simulink model "VirtualADAPT"  (Simulink 8.9)
settings: Solver=ode45  StartTime=0.0  StopTime=inf  RelTol=1e-3  EnableMultiTasking=on
PostLoadFcn:
  | FaultInjectionGUIHandle = FaultInjectionGUI;
InitFcn:
  | VirtualADAPTDataStructure
undefined symbols (not defined in the file or in its folder): ln
libraries: simulink x363
(part 20 of 26)

== system VirtualADAPT/VirtualADAPTv1/PowerStorage/Battery1Subsystem/SensorE142/R/Computing R ==
  In1 : Inport
  RNominal : Inport  Port=2
  Fault Profile : Inport  Port=3
  Step : Inport  Port=4
  Ramp : Inport  Port=5
  JuncDB : Inport  Port=6
  Bond : Inport  Port=7
  Junction Type : Inport  Port=8
  CalcCausality : Fcn  Expr=(u(1) == u(2) && u(3) ==1) || (u(1) !=u(2) && u(3) == 0)
  CalcOutput : Fcn  Expr=(u(1)*u(2)^u(3))
  MinusOne : Constant  Value=-1
  Mux1 : Mux  Inputs=3
  Mux2 : Mux  Inputs=3
  One : Constant
  Switch1 : MultiPortSwitch  SaturateOnIntegerOverflow=on  InputSameDT=on
  Switch2 : MultiPortSwitch  DataPortOrder=Zero-based contiguous  Inputs=2  zeroidx=on  SaturateOnIntegerOverflow=on  InputSameDT=on
  Out1 : Outport
  Out2 : Outport  Port=2
  wires:
  In1/1 -> Mux2/1
  Fault Profile/1 -> Switch1/1
  RNominal/1 -> Switch1/2
  Step/1 -> Switch1/3
  Ramp/1 -> Switch1/4
  Switch1/1 -> Out2/1, Mux2/2
  JuncDB/1 -> Mux1/1
  Bond/1 -> Mux1/2
  Junction Type/1 -> Mux1/3
  Mux1/1 -> CalcCausality/1
  CalcCausality/1 -> Switch2/1
  One/1 -> Switch2/2
  MinusOne/1 -> Switch2/3
  Switch2/1 -> Mux2/3
  Mux2/1 -> CalcOutput/1
  CalcOutput/1 -> Out1/1

== system VirtualADAPT/VirtualADAPTv1/PowerStorage/Battery1Subsystem/SensorISH136/MSe/Fault Inject ==
  Nominal : Inport
  Fault Profile : Inport  Port=2
  Step : Inport  Port=3
  Ramp : Inport  Port=4
  Bias : Inport  Port=5
  stuckAt : Inport  Port=6
  Switch1 : MultiPortSwitch  Inputs=5  SaturateOnIntegerOverflow=on  InputSameDT=on
  Out1 : Outport
  wires:
  Nominal/1 -> Switch1/2
  Fault Profile/1 -> Switch1/1
  Step/1 -> Switch1/3
  Ramp/1 -> Switch1/4
  Bias/1 -> Switch1/5
  stuckAt/1 -> Switch1/6
  Switch1/1 -> Out1/1

== system VirtualADAPT/VirtualADAPTv1/PowerStorage/Battery1Subsystem/SensorISH136/R/Computing R ==
  In1 : Inport
  RNominal : Inport  Port=2
  Fault Profile : Inport  Port=3
  Step : Inport  Port=4
  Ramp : Inport  Port=5
  JuncDB : Inport  Port=6
  Bond : Inport  Port=7
  Junction Type : Inport  Port=8
  CalcCausality : Fcn  Expr=(u(1) == u(2) && u(3) ==1) || (u(1) !=u(2) && u(3) == 0)
  CalcOutput : Fcn  Expr=(u(1)*u(2)^u(3))
  MinusOne : Constant  Value=-1
  Mux1 : Mux  Inputs=3
  Mux2 : Mux  Inputs=3
  One : Constant
  Switch1 : MultiPortSwitch  SaturateOnIntegerOverflow=on  InputSameDT=on
  Switch2 : MultiPortSwitch  DataPortOrder=Zero-based contiguous  Inputs=2  zeroidx=on  SaturateOnIntegerOverflow=on  InputSameDT=on
  Out1 : Outport
  Out2 : Outport  Port=2
  wires:
  In1/1 -> Mux2/1
  Fault Profile/1 -> Switch1/1
  RNominal/1 -> Switch1/2
  Step/1 -> Switch1/3
  Ramp/1 -> Switch1/4
  Switch1/1 -> Out2/1, Mux2/2
  JuncDB/1 -> Mux1/1
  Bond/1 -> Mux1/2
  Junction Type/1 -> Mux1/3
  Mux1/1 -> CalcCausality/1
  CalcCausality/1 -> Switch2/1
  One/1 -> Switch2/2
  MinusOne/1 -> Switch2/3
  Switch2/1 -> Mux2/3
  Mux2/1 -> CalcOutput/1
  CalcOutput/1 -> Out1/1

== system VirtualADAPT/VirtualADAPTv1/PowerStorage/Battery1Subsystem/SensorIT140/MSe/Fault Inject ==
  Nominal : Inport
  Fault Profile : Inport  Port=2
  Step : Inport  Port=3
  Ramp : Inport  Port=4
  Bias : Inport  Port=5
  stuckAt : Inport  Port=6
  Switch1 : MultiPortSwitch  Inputs=5  SaturateOnIntegerOverflow=on  InputSameDT=on
  Out1 : Outport
  wires:
  Nominal/1 -> Switch1/2
  Fault Profile/1 -> Switch1/1
  Step/1 -> Switch1/3
  Ramp/1 -> Switch1/4
  Bias/1 -> Switch1/5
  stuckAt/1 -> Switch1/6
  Switch1/1 -> Out1/1

== system VirtualADAPT/VirtualADAPTv1/PowerStorage/Battery1Subsystem/SensorIT140/R/Computing R ==
  In1 : Inport
  RNominal : Inport  Port=2
  Fault Profile : Inport  Port=3
  Step : Inport  Port=4
  Ramp : Inport  Port=5
  JuncDB : Inport  Port=6
  Bond : Inport  Port=7
  Junction Type : Inport  Port=8
  CalcCausality : Fcn  Expr=(u(1) == u(2) && u(3) ==1) || (u(1) !=u(2) && u(3) == 0)
  CalcOutput : Fcn  Expr=(u(1)*u(2)^u(3))
  MinusOne : Constant  Value=-1
  Mux1 : Mux  Inputs=3
  Mux2 : Mux  Inputs=3
  One : Constant
  Switch1 : MultiPortSwitch  SaturateOnIntegerOverflow=on  InputSameDT=on
  Switch2 : MultiPortSwitch  DataPortOrder=Zero-based contiguous  Inputs=2  zeroidx=on  SaturateOnIntegerOverflow=on  InputSameDT=on
  Out1 : Outport
  Out2 : Outport  Port=2
  wires:
  In1/1 -> Mux2/1
  Fault Profile/1 -> Switch1/1
  RNominal/1 -> Switch1/2
  Step/1 -> Switch1/3
  Ramp/1 -> Switch1/4
  Switch1/1 -> Out2/1, Mux2/2
  JuncDB/1 -> Mux1/1
  Bond/1 -> Mux1/2
  Junction Type/1 -> Mux1/3
  Mux1/1 -> CalcCausality/1
  CalcCausality/1 -> Switch2/1
  One/1 -> Switch2/2
  MinusOne/1 -> Switch2/3
  Switch2/1 -> Mux2/3
  Mux2/1 -> CalcOutput/1
  CalcOutput/1 -> Out1/1

== system VirtualADAPT/VirtualADAPTv1/PowerStorage/Battery1Subsystem/SensorTE128/MSe/Fault Inject ==
  Nominal : Inport
  Fault Profile : Inport  Port=2
  Step : Inport  Port=3
  Ramp : Inport  Port=4
  Bias : Inport  Port=5
  stuckAt : Inport  Port=6
  Switch1 : MultiPortSwitch  Inputs=5  SaturateOnIntegerOverflow=on  InputSameDT=on
  Out1 : Outport
  wires:
  Nominal/1 -> Switch1/2
  Fault Profile/1 -> Switch1/1
  Step/1 -> Switch1/3
  Ramp/1 -> Switch1/4
  Bias/1 -> Switch1/5
  stuckAt/1 -> Switch1/6
  Switch1/1 -> Out1/1

== system VirtualADAPT/VirtualADAPTv1/PowerStorage/Battery1Subsystem/SensorTE128/R/Computing R ==
  In1 : Inport
  RNominal : Inport  Port=2
  Fault Profile : Inport  Port=3
  Step : Inport  Port=4
  Ramp : Inport  Port=5
  JuncDB : Inport  Port=6
  Bond : Inport  Port=7
  Junction Type : Inport  Port=8
  CalcCausality : Fcn  Expr=(u(1) == u(2) && u(3) ==1) || (u(1) !=u(2) && u(3) == 0)
  CalcOutput : Fcn  Expr=(u(1)*u(2)^u(3))
  MinusOne : Constant  Value=-1
  Mux1 : Mux  Inputs=3
  Mux2 : Mux  Inputs=3
  One : Constant
  Switch1 : MultiPortSwitch  SaturateOnIntegerOverflow=on  InputSameDT=on
  Switch2 : MultiPortSwitch  DataPortOrder=Zero-based contiguous  Inputs=2  zeroidx=on  SaturateOnIntegerOverflow=on  InputSameDT=on
  Out1 : Outport
  Out2 : Outport  Port=2
  wires:
  In1/1 -> Mux2/1
  Fault Profile/1 -> Switch1/1
  RNominal/1 -> Switch1/2
  Step/1 -> Switch1/3
  Ramp/1 -> Switch1/4
  Switch1/1 -> Out2/1, Mux2/2
  JuncDB/1 -> Mux1/1
  Bond/1 -> Mux1/2
  Junction Type/1 -> Mux1/3
  Mux1/1 -> CalcCausality/1
  CalcCausality/1 -> Switch2/1
  One/1 -> Switch2/2
  MinusOne/1 -> Switch2/3
  Switch2/1 -> Mux2/3
  Mux2/1 -> CalcOutput/1
  CalcOutput/1 -> Out1/1

== system VirtualADAPT/VirtualADAPTv1/PowerStorage/Battery1Subsystem/SensorTE128/TempBias/Fault Inject ==
  Nominal : Inport
  Fault Profile : Inport  Port=2
  Step : Inport  Port=3
  Ramp : Inport  Port=4
  Bias : Inport  Port=5
  stuckAt : Inport  Port=6
  Switch1 : MultiPortSwitch  Inputs=5  SaturateOnIntegerOverflow=on  InputSameDT=on
  Out1 : Outport
  wires:
  Nominal/1 -> Switch1/2
  Fault Profile/1 -> Switch1/1
  Step/1 -> Switch1/3
  Ramp/1 -> Switch1/4
  Bias/1 -> Switch1/5
  stuckAt/1 -> Switch1/6
  Switch1/1 -> Out1/1

== system VirtualADAPT/VirtualADAPTv1/PowerStorage/Battery1Subsystem/SensorTE129/MSe/Fault Inject ==
  Nominal : Inport
  Fault Profile : Inport  Port=2
  Step : Inport  Port=3
  Ramp : Inport  Port=4
  Bias : Inport  Port=5
  stuckAt : Inport  Port=6
  Switch1 : MultiPortSwitch  Inputs=5  SaturateOnIntegerOverflow=on  InputSameDT=on
  Out1 : Outport
  wires:
  Nominal/1 -> Switch1/2
  Fault Profile/1 -> Switch1/1
  Step/1 -> Switch1/3
  Ramp/1 -> Switch1/4
  Bias/1 -> Switch1/5
  stuckAt/1 -> Switch1/6
  Switch1/1 -> Out1/1

== system VirtualADAPT/VirtualADAPTv1/PowerStorage/Battery1Subsystem/SensorTE129/R/Computing R ==
  In1 : Inport
  RNominal : Inport  Port=2
  Fault Profile : Inport  Port=3
  Step : Inport  Port=4
  Ramp : Inport  Port=5
  JuncDB : Inport  Port=6
  Bond : Inport  Port=7
  Junction Type : Inport  Port=8
  CalcCausality : Fcn  Expr=(u(1) == u(2) && u(3) ==1) || (u(1) !=u(2) && u(3) == 0)
  CalcOutput : Fcn  Expr=(u(1)*u(2)^u(3))
  MinusOne : Constant  Value=-1
  Mux1 : Mux  Inputs=3
  Mux2 : Mux  Inputs=3
  One : Constant
  Switch1 : MultiPortSwitch  SaturateOnIntegerOverflow=on  InputSameDT=on
  Switch2 : MultiPortSwitch  DataPortOrder=Zero-based contiguous  Inputs=2  zeroidx=on  SaturateOnIntegerOverflow=on  InputSameDT=on
  Out1 : Outport
  Out2 : Outport  Port=2
  wires:
  In1/1 -> Mux2/1
  Fault Profile/1 -> Switch1/1
  RNominal/1 -> Switch1/2
  Step/1 -> Switch1/3
  Ramp/1 -> Switch1/4
  Switch1/1 -> Out2/1, Mux2/2
  JuncDB/1 -> Mux1/1
  Bond/1 -> Mux1/2
  Junction Type/1 -> Mux1/3
  Mux1/1 -> CalcCausality/1
  CalcCausality/1 -> Switch2/1
  One/1 -> Switch2/2
  MinusOne/1 -> Switch2/3
  Switch2/1 -> Mux2/3
  Mux2/1 -> CalcOutput/1
  CalcOutput/1 -> Out1/1

== system VirtualADAPT/VirtualADAPTv1/PowerStorage/Battery1Subsystem/SensorTE129/TempBias/Fault Inject ==
  Nominal : Inport
  Fault Profile : Inport  Port=2
  Step : Inport  Port=3
  Ramp : Inport  Port=4
  Bias : Inport  Port=5
  stuckAt : Inport  Port=6
  Switch1 : MultiPortSwitch  Inputs=5  SaturateOnIntegerOverflow=on  InputSameDT=on
  Out1 : Outport
  wires:
  Nominal/1 -> Switch1/2
  Fault Profile/1 -> Switch1/1
  Step/1 -> Switch1/3
  Ramp/1 -> Switch1/4
  Bias/1 -> Switch1/5
  stuckAt/1 -> Switch1/6
  Switch1/1 -> Out1/1

== system VirtualADAPT/VirtualADAPTv1/PowerStorage/Battery2Subsystem/Battery2/Charge/C ==
  F : Inport
  Capacitance : Constant  Value=C
  Fault Inject : Subsystem
  Fault Profile : Constant  Value=FP
  InitialCondition : Constant  Value=IC
  Integrator : Integrator  InitialConditionSource=external
  Product1 : Product  SaturateOnIntegerOverflow=on  RndMeth=Zero  InputSameDT=on  OutDataTypeStr=Inherit: Same as first input
  Product2 : Product  SaturateOnIntegerOverflow=on  RndMeth=Zero  InputSameDT=on  OutDataTypeStr=Inherit: Same as first input
  Ramp : simulink/Sources/Ramp  slope=FM  start=FT  X0=C
  Reciprocal : Math  Operator=reciprocal  SaturateOnIntegerOverflow=on
  Step : Step  Time=FT  Before=C  After=(1 + FM)*C  SampleTime=0
  E : Outport
  C : Outport  Port=2
  q : Outport  Port=3
  wires:
  F/1 -> Integrator/1
  InitialCondition/1 -> Product1/1
  Capacitance/1 -> Fault Inject/1(Nominal), Product1/2
  Product1/1 -> Integrator/2
  Integrator/1 -> Product2/1, q/1
  Fault Inject/1(Out1) -> Reciprocal/1, C/1
  Reciprocal/1 -> Product2/2
  Fault Profile/1 -> Fault Inject/2(Fault Profile)
  Step/1 -> Fault Inject/3(Step)
  Ramp/1 -> Fault Inject/4(Ramp)
  Product2/1 -> E/1

== system VirtualADAPT/VirtualADAPTv1/PowerStorage/Battery2Subsystem/Battery2/Charge/ComputeDOC ==
  u(1) : Inport
  u(2) : Inport  Port=2
  u(3) : Inport  Port=3
  Function : Fcn  Expr=1-(2765360-u(1))/(1.33*270720*(1-u(3)/(-35))^0.642/(1+(1.33-1)*(u(2)/5)^0.61))
  Mux : Mux  Inputs=3
  y(1) : Outport
  wires:
  u(1)/1 -> Mux/1
  u(2)/1 -> Mux/2
  u(3)/1 -> Mux/3
  Mux/1 -> Function/1
  Function/1 -> y(1)/1

== system VirtualADAPT/VirtualADAPTv1/PowerStorage/Battery2Subsystem/Battery2/Charge/ComputeSOC ==
  u(1) : Inport
  u(2) : Inport  Port=2
  Function : Fcn  Expr=1-(2765360-u(2))/(1.33*270720*(1-u(1)/(-35))^0.642)
  Mux : Mux  Inputs=2
  y(1) : Outport
  wires:
  u(1)/1 -> Mux/1
  u(2)/1 -> Mux/2
  Mux/1 -> Function/1
  Function/1 -> y(1)/1

== system VirtualADAPT/VirtualADAPTv1/PowerStorage/Battery2Subsystem/Battery2/Charge/Function ==
  u(1) : Inport
  Function : Fcn  Expr=u(1)
  Mux : Mux  Inputs=1
  y(1) : Outport
  wires:
  u(1)/1 -> Mux/1
  Mux/1 -> Function/1
  Function/1 -> y(1)/1

== system VirtualADAPT/VirtualADAPTv1/PowerStorage/Battery2Subsystem/Battery2/Charge/IbattNegative ==
  u(1) : Inport
  Function : Fcn  Expr=u(1)<0
  Mux : Mux  Inputs=1
  One : Constant
  Switch : Switch  Criteria=u2 ~= 0  SaturateOnIntegerOverflow=on  InputSameDT=on
  Zero : Constant  Value=0
  y(1) : Outport
  wires:
  u(1)/1 -> Mux/1
  Mux/1 -> Function/1
  Function/1 -> Switch/2
  One/1 -> Switch/1
  Zero/1 -> Switch/3
  Switch/1 -> y(1)/1

== system VirtualADAPT/VirtualADAPTv1/PowerStorage/Battery2Subsystem/Battery2/Charge/Imod ==
  u(1) : Inport
  u(2) : Inport  Port=2
  u(3) : Inport  Port=3
  Adder : Sum  Inputs=-  SaturateOnIntegerOverflow=on  InputSameDT=on  OutDataTypeStr=Inherit: Same as first input
  Gain : Gain  Gain=-1  SaturateOnIntegerOverflow=on  OutDataTypeStr=Inherit: Same as input  ParamDataTypeStr=Inherit: Same as input
  Mux : Mux  Inputs=3
  OffGuard : Fcn  Expr=(u(3))
  OnGuard : Fcn  Expr=(!u(3))
  Product1 : Product  SaturateOnIntegerOverflow=on  RndMeth=Zero  InputSameDT=on  OutDataTypeStr=Inherit: Same as first input
  Product2 : Product  SaturateOnIntegerOverflow=on  RndMeth=Zero  InputSameDT=on  OutDataTypeStr=Inherit: Same as first input
  determineState : Subsystem
  y(1) : Outport
  y(2) : Outport  Port=2
  O : Outport  Port=3
  State : Outport  Port=4
  wires:
  Adder/1 -> Gain/1
  u(3)/1 -> Mux/3
  Mux/1 -> OnGuard/1, OffGuard/1
  OnGuard/1 -> determineState/1(In1)
  OffGuard/1 -> determineState/2(In2)
  u(2)/1 -> Product1/1
  determineState/1(Out1) -> Product1/2, Product2/2, State/1
  Product1/1 -> O/1, y(1)/1
  Gain/1 -> Product2/1
  u(1)/1 -> Adder/1
  Product2/1 -> y(2)/1

== system VirtualADAPT/VirtualADAPTv1/PowerStorage/Battery2Subsystem/Battery2/Charge/Sf ==
  E : Inport
  Sf : Inport  Port=2
  Bias : Step  Time=FT  After=FM  SampleTime=0
  Fault Inject : Subsystem
  Fault Profile : Constant  Value=FP
  Mult2 : Product  SaturateOnIntegerOverflow=on  RndMeth=Zero  InputSameDT=on  OutDataTypeStr=Inherit: Same as first input
  Multiply : Product  SaturateOnIntegerOverflow=on  RndMeth=Zero  InputSameDT=on  OutDataTypeStr=Inherit: Same as first input
  Ramp : simulink/Sources/Ramp  slope=FM  start=FT  X0=0
  Step : Step  Time=FT  Before=1  After=(1 + FM)  SampleTime=0
  StuckAt : Step  Time=FT  Before=1  After=0  SampleTime=0
  StuckAt2 : Step  Time=FT  After=FM  SampleTime=0
  Sum : Sum  SaturateOnIntegerOverflow=on  InputSameDT=on  OutDataTypeStr=Inherit: Same as first input
  Sum2 : Sum  SaturateOnIntegerOverflow=on  InputSameDT=on  OutDataTypeStr=Inherit: Same as first input
  Sum3 : Sum  SaturateOnIntegerOverflow=on  InputSameDT=on  OutDataTypeStr=Inherit: Same as first input
  F : Outport
  wires:
  Fault Inject/1(Out1) -> F/1
  Sf/1 -> Fault Inject/1(Nominal), Multiply/1, Sum/1, Sum2/1, Mult2/1
  Fault Profile/1 -> Fault Inject/2(Fault Profile)
  Step/1 -> Multiply/2
  Ramp/1 -> Sum/2
  Multiply/1 -> Fault Inject/3(Step)
  Sum/1 -> Fault Inject/4(Ramp)
  Bias/1 -> Sum2/2
  Sum2/1 -> Fault Inject/5(Bias)
  StuckAt/1 -> Mult2/2
  Mult2/1 -> Sum3/1
  StuckAt2/1 -> Sum3/2
  Sum3/1 -> Fault Inject/6(stuckAt)

== system VirtualADAPT/VirtualADAPTv1/PowerStorage/Battery2Subsystem/Battery2/Charge/ZeroJunction ==
  u(1) : Inport
  u(2) : Inport  Port=2
  Adder : Sum  Inputs=+  SaturateOnIntegerOverflow=on  InputSameDT=on  OutDataTypeStr=Inherit: Same as first input
  Gain : Gain  SaturateOnIntegerOverflow=on  OutDataTypeStr=Inherit: Same as input  ParamDataTypeStr=Inherit: Same as input
  y(1) : Outport
  y(2) : Outport  Port=2
  O : Outport  Port=3
  wires:
  Adder/1 -> Gain/1
  u(2)/1 -> y(1)/1, O/1
  u(1)/1 -> Adder/1
  Gain/1 -> y(2)/1

== system VirtualADAPT/VirtualADAPTv1/PowerStorage/Battery2Subsystem/Battery2/CircuitEquivalent/BatteryConnected ==
  Constant : Constant  Value=Constant
  Fault Inject : Subsystem
  Fault Profile : Constant  Value=FP
  Ramp : simulink/Sources/Ramp  slope=FM  start=FT  X0=Constant
  Step : Step  Time=FT  Before=Constant  After=(1 + FM)*Constant  SampleTime=0
  C : Outport
  wires:
  Fault Inject/1(Out1) -> C/1
  Constant/1 -> Fault Inject/1(Nominal)
  Fault Profile/1 -> Fault Inject/2(Fault Profile)
  Step/1 -> Fault Inject/3(Step)
  Ramp/1 -> Fault Inject/4(Ramp)

== system VirtualADAPT/VirtualADAPTv1/PowerStorage/Battery2Subsystem/Battery2/CircuitEquivalent/Bug ==
  u(1) : Inport
  u(2) : Inport  Port=2
  Adder : Sum  Inputs=+  SaturateOnIntegerOverflow=on  InputSameDT=on  OutDataTypeStr=Inherit: Same as first input
  Gain : Gain  SaturateOnIntegerOverflow=on  OutDataTypeStr=Inherit: Same as input  ParamDataTypeStr=Inherit: Same as input
  y(1) : Outport
  y(2) : Outport  Port=2
  O : Outport  Port=3
  wires:
  Adder/1 -> Gain/1
  Gain/1 -> y(1)/1
  u(1)/1 -> y(2)/1, O/1
  u(2)/1 -> Adder/1

== system VirtualADAPT/VirtualADAPTv1/PowerStorage/Battery2Subsystem/Battery2/CircuitEquivalent/C0 ==
  F : Inport
  Capacitance : Constant  Value=C
  Data Type Conversion : DataTypeConversion  OutDataTypeStr=boolean
  Detect Change : simulink/Logic and Bit Operations/Detect Change  vinit=0  InputProcessing=Inherited  OutDataTypeStr=uint8
  Fault Inject : Subsystem
  Fault Profile : Constant  Value=FP
  InitialCondition : Constant  Value=IC
  Integrator : Integrator  ExternalReset=either  InitialConditionSource=external
  Product1 : Product  SaturateOnIntegerOverflow=on  RndMeth=Zero  InputSameDT=on  OutDataTypeStr=Inherit: Same as first input
  Product2 : Product  SaturateOnIntegerOverflow=on  RndMeth=Zero  InputSameDT=on  OutDataTypeStr=Inherit: Same as first input
  Ramp : simulink/Sources/Ramp  slope=FM  start=FT  X0=C
  Reciprocal : Math  Operator=reciprocal  SaturateOnIntegerOverflow=on
  Step : Step  Time=FT  Before=C  After=(1 + FM)*C  SampleTime=0
  E : Outport
  C : Outport  Port=2
  q : Outport  Port=3
  wires:
  F/1 -> Integrator/1
  InitialCondition/1 -> Product1/1, Detect Change/1
  Capacitance/1 -> Fault Inject/1(Nominal), Product1/2
  Product1/1 -> Integrator/3
  Integrator/1 -> Product2/1, q/1
  Fault Inject/1(Out1) -> Reciprocal/1, C/1
  Reciprocal/1 -> Product2/2
  Fault Profile/1 -> Fault Inject/2(Fault Profile)
  Step/1 -> Fault Inject/3(Step)
  Ramp/1 -> Fault Inject/4(Ramp)
  Product2/1 -> E/1
  Detect Change/1 -> Data Type Conversion/1
  Data Type Conversion/1 -> Integrator/2

== system VirtualADAPT/VirtualADAPTv1/PowerStorage/Battery2Subsystem/Battery2/CircuitEquivalent/C1 ==
  F : Inport
  Capacitance : Constant  Value=C
  Fault Inject : Subsystem
  Fault Profile : Constant  Value=FP
  InitialCondition : Constant  Value=IC
  Integrator : Integrator  InitialConditionSource=external
  Product1 : Product  SaturateOnIntegerOverflow=on  RndMeth=Zero  InputSameDT=on  OutDataTypeStr=Inherit: Same as first input
  Product2 : Product  SaturateOnIntegerOverflow=on  RndMeth=Zero  InputSameDT=on  OutDataTypeStr=Inherit: Same as first input
  Ramp : simulink/Sources/Ramp  slope=FM  start=FT  X0=C
  Reciprocal : Math  Operator=reciprocal  SaturateOnIntegerOverflow=on
  Step : Step  Time=FT  Before=C  After=(1 + FM)*C  SampleTime=0
  E : Outport
  C : Outport  Port=2
  q : Outport  Port=3
  wires:
  F/1 -> Integrator/1
  InitialCondition/1 -> Product1/1
  Capacitance/1 -> Fault Inject/1(Nominal), Product1/2
  Product1/1 -> Integrator/2
  Integrator/1 -> Product2/1, q/1
  Fault Inject/1(Out1) -> Reciprocal/1, C/1
  Reciprocal/1 -> Product2/2
  Fault Profile/1 -> Fault Inject/2(Fault Profile)
  Step/1 -> Fault Inject/3(Step)
  Ramp/1 -> Fault Inject/4(Ramp)
  Product2/1 -> E/1

== system VirtualADAPT/VirtualADAPTv1/PowerStorage/Battery2Subsystem/Battery2/CircuitEquivalent/C2 ==
  F : Inport
  Capacitance : Constant  Value=C
  Fault Inject : Subsystem
  Fault Profile : Constant  Value=FP
  InitialCondition : Constant  Value=IC
  Integrator : Integrator  InitialConditionSource=external
  Product1 : Product  SaturateOnIntegerOverflow=on  RndMeth=Zero  InputSameDT=on  OutDataTypeStr=Inherit: Same as first input
  Product2 : Product  SaturateOnIntegerOverflow=on  RndMeth=Zero  InputSameDT=on  OutDataTypeStr=Inherit: Same as first input
  Ramp : simulink/Sources/Ramp  slope=FM  start=FT  X0=C
  Reciprocal : Math  Operator=reciprocal  SaturateOnIntegerOverflow=on
  Step : Step  Time=FT  Before=C  After=(1 + FM)*C  SampleTime=0
  E : Outport
  C : Outport  Port=2
  q : Outport  Port=3
  wires:
  F/1 -> Integrator/1
  InitialCondition/1 -> Product1/1
  Capacitance/1 -> Fault Inject/1(Nominal), Product1/2
  Product1/1 -> Integrator/2
  Integrator/1 -> Product2/1, q/1
  Fault Inject/1(Out1) -> Reciprocal/1, C/1
  Reciprocal/1 -> Product2/2
  Fault Profile/1 -> Fault Inject/2(Fault Profile)
  Step/1 -> Fault Inject/3(Step)
  Ramp/1 -> Fault Inject/4(Ramp)
  Product2/1 -> E/1

== system VirtualADAPT/VirtualADAPTv1/PowerStorage/Battery2Subsystem/Battery2/CircuitEquivalent/C3 ==
  F : Inport
  Capacitance : Constant  Value=C
  Fault Inject : Subsystem
  Fault Profile : Constant  Value=FP
  InitialCondition : Constant  Value=IC
  Integrator : Integrator  InitialConditionSource=external
  Product1 : Product  SaturateOnIntegerOverflow=on  RndMeth=Zero  InputSameDT=on  OutDataTypeStr=Inherit: Same as first input
  Product2 : Product  SaturateOnIntegerOverflow=on  RndMeth=Zero  InputSameDT=on  OutDataTypeStr=Inherit: Same as first input
  Ramp : simulink/Sources/Ramp  slope=FM  start=FT  X0=C
  Reciprocal : Math  Operator=reciprocal  SaturateOnIntegerOverflow=on
  Step : Step  Time=FT  Before=C  After=(1 + FM)*C  SampleTime=0
  E : Outport
  C : Outport  Port=2
  q : Outport  Port=3
  wires:
  F/1 -> Integrator/1
  InitialCondition/1 -> Product1/1
  Capacitance/1 -> Fault Inject/1(Nominal), Product1/2
  Product1/1 -> Integrator/2
  Integrator/1 -> Product2/1, q/1
  Fault Inject/1(Out1) -> Reciprocal/1, C/1
  Reciprocal/1 -> Product2/2
  Fault Profile/1 -> Fault Inject/2(Fault Profile)
  Step/1 -> Fault Inject/3(Step)
  Ramp/1 -> Fault Inject/4(Ramp)
  Product2/1 -> E/1

== system VirtualADAPT/VirtualADAPTv1/PowerStorage/Battery2Subsystem/Battery2/CircuitEquivalent/ComputePower ==
  u(1) : Inport
  u(2) : Inport  Port=2
  Function : Fcn  Expr=abs(u(1)*u(2))
  Mux : Mux  Inputs=2
  y(1) : Outport
  wires:
  u(1)/1 -> Mux/1
  u(2)/1 -> Mux/2
  Mux/1 -> Function/1
  Function/1 -> y(1)/1

== system VirtualADAPT/VirtualADAPTv1/PowerStorage/Battery2Subsystem/Battery2/CircuitEquivalent/I ==
  u(1) : Inport
  u(2) : Inport  Port=2
  u(3) : Inport  Port=3
  Adder : Sum  Inputs=+  SaturateOnIntegerOverflow=on  InputSameDT=on  OutDataTypeStr=Inherit: Same as first input
  Gain : Gain  SaturateOnIntegerOverflow=on  OutDataTypeStr=Inherit: Same as input  ParamDataTypeStr=Inherit: Same as input
  Mux : Mux  Inputs=3
  OffGuard : Fcn  Expr=(!u(3))
  OnGuard : Fcn  Expr=(u(3))
  Product1 : Product  SaturateOnIntegerOverflow=on  RndMeth=Zero  InputSameDT=on  OutDataTypeStr=Inherit: Same as first input
  Product2 : Product  SaturateOnIntegerOverflow=on  RndMeth=Zero  InputSameDT=on  OutDataTypeStr=Inherit: Same as first input
  determineState : Subsystem
  y(1) : Outport
  y(2) : Outport  Port=2
  O : Outport  Port=3
  State : Outport  Port=4
  wires:
  Adder/1 -> Gain/1
  u(3)/1 -> Mux/3
  Mux/1 -> OnGuard/1, OffGuard/1
  OnGuard/1 -> determineState/1(In1)
  OffGuard/1 -> determineState/2(In2)
  u(2)/1 -> Product1/1
  determineState/1(Out1) -> Product1/2, Product2/2, State/1
  Product1/1 -> O/1, y(1)/1
  Gain/1 -> Product2/1
  u(1)/1 -> Adder/1
  Product2/1 -> y(2)/1

== system VirtualADAPT/VirtualADAPTv1/PowerStorage/Battery2Subsystem/Battery2/CircuitEquivalent/InternalCurrent ==
  u(1) : Inport
  u(2) : Inport  Port=2
  u(3) : Inport  Port=3
  u(4) : Inport  Port=4
  u(5) : Inport  Port=5
  Adder : Sum  Inputs=----  SaturateOnIntegerOverflow=on  InputSameDT=on  OutDataTypeStr=Inherit: Same as first input
  Gain : Gain  Gain=-1  SaturateOnIntegerOverflow=on  OutDataTypeStr=Inherit: Same as input  ParamDataTypeStr=Inherit: Same as input
  y(1) : Outport
  y(2) : Outport  Port=2
  y(3) : Outport  Port=3
  y(4) : Outport  Port=4
  y(5) : Outport  Port=5
  O : Outport  Port=6
  wires:
  Adder/1 -> Gain/1
  Gain/1 -> y(1)/1
  u(1)/1 -> y(2)/1, y(3)/1, y(4)/1, y(5)/1, O/1
  u(2)/1 -> Adder/1
  u(3)/1 -> Adder/2
  u(4)/1 -> Adder/3
  u(5)/1 -> Adder/4

== system VirtualADAPT/VirtualADAPTv1/PowerStorage/Battery2Subsystem/Battery2/CircuitEquivalent/R1 ==
  In : Inport
  RI : Inport  Port=2
  Bond : Constant
  Computing R : Subsystem
  Fault Profile : Constant  Value=FP
  JuncDB : Constant  Value=3
  Junction Type : Constant  Value=0
  Multiply : Product  SaturateOnIntegerOverflow=on  RndMeth=Zero  InputSameDT=on  OutDataTypeStr=Inherit: Same as first input
  Ramp : simulink/Sources/Ramp  slope=FM  start=FT  X0=0
  Step : Step  Time=FT  Before=1  After=(1 + FM)  SampleTime=0
  Sum : Sum  SaturateOnIntegerOverflow=on  InputSameDT=on  OutDataTypeStr=Inherit: Same as first input
  Out : Outport
  RO : Outport  Port=2
  wires:
  Computing R/1(Out1) -> Out/1
  In/1 -> Computing R/1(In1)
  RI/1 -> Computing R/2(RNominal), Multiply/1, Sum/1
  Fault Profile/1 -> Computing R/3(Fault Profile)
  Step/1 -> Multiply/2
  Ramp/1 -> Sum/2
  JuncDB/1 -> Computing R/6(JuncDB)
  Bond/1 -> Computing R/7(Bond)
  Junction Type/1 -> Computing R/8(Junction Type)
  Computing R/2(Out2) -> RO/1
  Multiply/1 -> Computing R/4(Step)
  Sum/1 -> Computing R/5(Ramp)

== system VirtualADAPT/VirtualADAPTv1/PowerStorage/Battery2Subsystem/Battery2/CircuitEquivalent/R1 Mod ==
  u(1) : Inport
  Function : Fcn  Expr=2.2287e-014+0.063059*(u(1))
  Mux : Mux  Inputs=1
  y(1) : Outport
  wires:
  u(1)/1 -> Mux/1
  Mux/1 -> Function/1
  Function/1 -> y(1)/1

== system VirtualADAPT/VirtualADAPTv1/PowerStorage/Battery2Subsystem/Battery2/CircuitEquivalent/R2 ==
  In : Inport
  RI : Inport  Port=2
  Bond : Constant
  Computing R : Subsystem
  Fault Profile : Constant  Value=FP
  JuncDB : Constant  Value=2
  Junction Type : Constant  Value=0
  Multiply : Product  SaturateOnIntegerOverflow=on  RndMeth=Zero  InputSameDT=on  OutDataTypeStr=Inherit: Same as first input
  Ramp : simulink/Sources/Ramp  slope=FM  start=FT  X0=0
  Step : Step  Time=FT  Before=1  After=(1 + FM)  SampleTime=0
  Sum : Sum  SaturateOnIntegerOverflow=on  InputSameDT=on  OutDataTypeStr=Inherit: Same as first input
  Out : Outport
  RO : Outport  Port=2
  wires:
  Computing R/1(Out1) -> Out/1
  In/1 -> Computing R/1(In1)
  RI/1 -> Computing R/2(RNominal), Multiply/1, Sum/1
  Fault Profile/1 -> Computing R/3(Fault Profile)
  Step/1 -> Multiply/2
  Ramp/1 -> Sum/2
  JuncDB/1 -> Computing R/6(JuncDB)
  Bond/1 -> Computing R/7(Bond)
  Junction Type/1 -> Computing R/8(Junction Type)
  Computing R/2(Out2) -> RO/1
  Multiply/1 -> Computing R/4(Step)
  Sum/1 -> Computing R/5(Ramp)

== system VirtualADAPT/VirtualADAPTv1/PowerStorage/Battery2Subsystem/Battery2/CircuitEquivalent/R2 Mod ==
  u(1) : Inport
  Function : Fcn  Expr=-0.0080551*ln(u(1))
  Mux : Mux  Inputs=1
  y(1) : Outport
  wires:
  u(1)/1 -> Mux/1
  Mux/1 -> Function/1
  Function/1 -> y(1)/1

== system VirtualADAPT/VirtualADAPTv1/PowerStorage/Battery2Subsystem/Battery2/CircuitEquivalent/R3 ==
  In : Inport
  RI : Inport  Port=2
  Bond : Constant
  Computing R : Subsystem
  Fault Profile : Constant  Value=FP
  JuncDB : Constant  Value=2
  Junction Type : Constant  Value=0
  Multiply : Product  SaturateOnIntegerOverflow=on  RndMeth=Zero  InputSameDT=on  OutDataTypeStr=Inherit: Same as first input
  Ramp : simulink/Sources/Ramp  slope=FM  start=FT  X0=0
  Step : Step  Time=FT  Before=1  After=(1 + FM)  SampleTime=0
  Sum : Sum  SaturateOnIntegerOverflow=on  InputSameDT=on  OutDataTypeStr=Inherit: Same as first input
  Out : Outport
  RO : Outport  Port=2
  wires:
  Computing R/1(Out1) -> Out/1
  In/1 -> Computing R/1(In1)
  RI/1 -> Computing R/2(RNominal), Multiply/1, Sum/1
  Fault Profile/1 -> Computing R/3(Fault Profile)
  Step/1 -> Multiply/2
  Ramp/1 -> Sum/2
  JuncDB/1 -> Computing R/6(JuncDB)
  Bond/1 -> Computing R/7(Bond)
  Junction Type/1 -> Computing R/8(Junction Type)
  Computing R/2(Out2) -> RO/1
  Multiply/1 -> Computing R/4(Step)
  Sum/1 -> Computing R/5(Ramp)

== system VirtualADAPT/VirtualADAPTv1/PowerStorage/Battery2Subsystem/Battery2/CircuitEquivalent/R3 Mod ==
  u(1) : Inport
  u(2) : Inport  Port=2
  Function : Fcn  Expr=0.25702*exp(-1.8187*(1-(u(2))))/(1+exp(0.20694*(u(1))))
  Mux : Mux  Inputs=2
  y(1) : Outport
  wires:
  u(1)/1 -> Mux/1
  u(2)/1 -> Mux/2
  Mux/1 -> Function/1
  Function/1 -> y(1)/1

== system VirtualADAPT/VirtualADAPTv1/PowerStorage/Battery2Subsystem/Battery2/CircuitEquivalent/RpDischarge ==
  In : Inport
  Bond : Constant  Value=2
  Computing R : Subsystem
  Fault Profile : Constant  Value=FP
  JuncDB : Constant
  Junction Type : Constant  Value=0
  Ramp : simulink/Sources/Ramp  slope=FM  start=FT  X0=R
  Resistor Value : Constant  Value=R
  Step : Step  Time=FT  Before=R  After=(1 + FM)*R  SampleTime=0
  Out : Outport
  R : Outport  Port=2
  wires:
  Computing R/1(Out1) -> Out/1
  In/1 -> Computing R/1(In1)
  Resistor Value/1 -> Computing R/2(RNominal)
  Fault Profile/1 -> Computing R/3(Fault Profile)
  Step/1 -> Computing R/4(Step)
  Ramp/1 -> Computing R/5(Ramp)
  JuncDB/1 -> Computing R/6(JuncDB)
  Bond/1 -> Computing R/7(Bond)
  Junction Type/1 -> Computing R/8(Junction Type)
  Computing R/2(Out2) -> R/1

== system VirtualADAPT/VirtualADAPTv1/PowerStorage/Battery2Subsystem/Battery2/CircuitEquivalent/V ==
  u(1) : Inport
  u(2) : Inport  Port=2
  u(3) : Inport  Port=3
  Adder : Sum  Inputs=-  SaturateOnIntegerOverflow=on  InputSameDT=on  OutDataTypeStr=Inherit: Same as first input
  Gain : Gain  Gain=-1  SaturateOnIntegerOverflow=on  OutDataTypeStr=Inherit: Same as input  ParamDataTypeStr=Inherit: Same as input
  Mux : Mux  Inputs=3
  OffGuard : Fcn  Expr=(!u(3))
  OnGuard : Fcn  Expr=(u(3))
  Product1 : Product  SaturateOnIntegerOverflow=on  RndMeth=Zero  InputSameDT=on  OutDataTypeStr=Inherit: Same as first input
  Product2 : Product  SaturateOnIntegerOverflow=on  RndMeth=Zero  InputSameDT=on  OutDataTypeStr=Inherit: Same as first input
  determineState : Subsystem
  y(1) : Outport
  y(2) : Outport  Port=2
  O : Outport  Port=3
  State : Outport  Port=4
  wires:
  Adder/1 -> Gain/1
  u(3)/1 -> Mux/3
  Mux/1 -> OnGuard/1, OffGuard/1
  OnGuard/1 -> determineState/1(In1)
  OffGuard/1 -> determineState/2(In2)
  u(1)/1 -> Product1/1
  determineState/1(Out1) -> Product1/2, Product2/2, State/1
  Product1/1 -> O/1, y(2)/1
  Gain/1 -> Product2/1
  Product2/1 -> y(1)/1
  u(2)/1 -> Adder/1

== system VirtualADAPT/VirtualADAPTv1/PowerStorage/Battery2Subsystem/Battery2/CircuitEquivalent/Vp ==
  u(1) : Inport
  u(2) : Inport  Port=2
  u(3) : Inport  Port=3
  Adder : Sum  Inputs=--  SaturateOnIntegerOverflow=on  InputSameDT=on  OutDataTypeStr=Inherit: Same as first input
  Gain : Gain  SaturateOnIntegerOverflow=on  OutDataTypeStr=Inherit: Same as input  ParamDataTypeStr=Inherit: Same as input
  y(1) : Outport
  y(2) : Outport  Port=2
  y(3) : Outport  Port=3
  O : Outport  Port=4
  wires:
  Adder/1 -> Gain/1
  Gain/1 -> y(1)/1
  u(1)/1 -> y(2)/1, y(3)/1, O/1
  u(2)/1 -> Adder/1
  u(3)/1 -> Adder/2

== system VirtualADAPT/VirtualADAPTv1/PowerStorage/Battery2Subsystem/Battery2/CircuitEquivalent/v1 ==
  u(1) : Inport
  u(2) : Inport  Port=2
  u(3) : Inport  Port=3
  Adder : Sum  Inputs=-+  SaturateOnIntegerOverflow=on  InputSameDT=on  OutDataTypeStr=Inherit: Same as first input
  Gain : Gain  SaturateOnIntegerOverflow=on  OutDataTypeStr=Inherit: Same as input  ParamDataTypeStr=Inherit: Same as input
  y(1) : Outport
  y(2) : Outport  Port=2
  y(3) : Outport  Port=3
  O : Outport  Port=4
  wires:
  Adder/1 -> Gain/1
  u(3)/1 -> y(1)/1, y(2)/1, O/1
  u(1)/1 -> Adder/1
  u(2)/1 -> Adder/2
  Gain/1 -> y(3)/1

== system VirtualADAPT/VirtualADAPTv1/PowerStorage/Battery2Subsystem/Battery2/CircuitEquivalent/v2 ==
  u(1) : Inport
  u(2) : Inport  Port=2
  u(3) : Inport  Port=3
  Adder : Sum  Inputs=-+  SaturateOnIntegerOverflow=on  InputSameDT=on  OutDataTypeStr=Inherit: Same as first input
  Gain : Gain  SaturateOnIntegerOverflow=on  OutDataTypeStr=Inherit: Same as input  ParamDataTypeStr=Inherit: Same as input
  y(1) : Outport
  y(2) : Outport  Port=2
  y(3) : Outport  Port=3
  O : Outport  Port=4
  wires:
  Adder/1 -> Gain/1
  u(2)/1 -> y(1)/1, y(3)/1, O/1
  u(1)/1 -> Adder/1
  Gain/1 -> y(2)/1
  u(3)/1 -> Adder/2

== system VirtualADAPT/VirtualADAPTv1/PowerStorage/Battery2Subsystem/Battery2/CircuitEquivalent/v3 ==
  u(1) : Inport
  u(2) : Inport  Port=2
  u(3) : Inport  Port=3
  Adder : Sum  Inputs=-+  SaturateOnIntegerOverflow=on  InputSameDT=on  OutDataTypeStr=Inherit: Same as first input
  Gain : Gain  SaturateOnIntegerOverflow=on  OutDataTypeStr=Inherit: Same as input  ParamDataTypeStr=Inherit: Same as input
  y(1) : Outport
  y(2) : Outport  Port=2
  y(3) : Outport  Port=3
  O : Outport  Port=4
  wires:
  Adder/1 -> Gain/1
  u(2)/1 -> y(1)/1, y(3)/1, O/1
  u(1)/1 -> Adder/1
  Gain/1 -> y(2)/1
  u(3)/1 -> Adder/2

== system VirtualADAPT/VirtualADAPTv1/PowerStorage/Battery2Subsystem/Battery2/TemperatureModel/AmbTemp ==
  F : Inport
  Se : Inport  Port=2
  Bias : Step  Time=FT  After=FM  SampleTime=0
  Fault Inject : Subsystem
  Fault Profile : Constant  Value=FP
  Mult2 : Product  SaturateOnIntegerOverflow=on  RndMeth=Zero  InputSameDT=on  OutDataTypeStr=Inherit: Same as first input
  Multiply : Product  SaturateOnIntegerOverflow=on  RndMeth=Zero  InputSameDT=on  OutDataTypeStr=Inherit: Same as first input
  Ramp : simulink/Sources/Ramp  slope=FM  start=FT  X0=0
  Step : Step  Time=FT  Before=1  After=(1 + FM)  SampleTime=0
  StuckAt : Step  Time=FT  Before=1  After=0  SampleTime=0
  StuckAt2 : Step  Time=FT  After=FM  SampleTime=0
  Sum : Sum  SaturateOnIntegerOverflow=on  InputSameDT=on  OutDataTypeStr=Inherit: Same as first input
  Sum2 : Sum  SaturateOnIntegerOverflow=on  InputSameDT=on  OutDataTypeStr=Inherit: Same as first input
  Sum3 : Sum  SaturateOnIntegerOverflow=on  InputSameDT=on  OutDataTypeStr=Inherit: Same as first input
  E : Outport
  wires:
  Fault Inject/1(Out1) -> E/1
  Se/1 -> Fault Inject/1(Nominal), Multiply/1, Sum/1, Sum2/1, Mult2/1
  Fault Profile/1 -> Fault Inject/2(Fault Profile)
  Step/1 -> Multiply/2
  Ramp/1 -> Sum/2
  Multiply/1 -> Fault Inject/3(Step)
  Sum/1 -> Fault Inject/4(Ramp)
  Bias/1 -> Sum2/2
  Sum2/1 -> Fault Inject/5(Bias)
  StuckAt/1 -> Mult2/2
  Mult2/1 -> Sum3/1
  StuckAt2/1 -> Sum3/2
  Sum3/1 -> Fault Inject/6(stuckAt)

== system VirtualADAPT/VirtualADAPTv1/PowerStorage/Battery2Subsystem/Battery2/TemperatureModel/Ctheta ==
  F : Inport
  Capacitance : Constant  Value=C
  Data Type Conversion : DataTypeConversion  OutDataTypeStr=boolean
  Detect Change : simulink/Logic and Bit Operations/Detect Change  vinit=0  InputProcessing=Inherited  OutDataTypeStr=uint8
  Fault Inject : Subsystem
  Fault Profile : Constant  Value=FP
  InitialCondition : Constant  Value=IC
  Integrator : Integrator  ExternalReset=either  InitialConditionSource=external
  Product1 : Product  SaturateOnIntegerOverflow=on  RndMeth=Zero  InputSameDT=on  OutDataTypeStr=Inherit: Same as first input
  Product2 : Product  SaturateOnIntegerOverflow=on  RndMeth=Zero  InputSameDT=on  OutDataTypeStr=Inherit: Same as first input
  Ramp : simulink/Sources/Ramp  slope=FM  start=FT  X0=C
  Reciprocal : Math  Operator=reciprocal  SaturateOnIntegerOverflow=on
  Saturation : Saturate  UpperLimit=3e+006  LowerLimit=1e+005
  Step : Step  Time=FT  Before=C  After=(1 + FM)*C  SampleTime=0
  E : Outport
  C : Outport  Port=2
  q : Outport  Port=3
  wires:
  F/1 -> Integrator/1
  InitialCondition/1 -> Product1/1, Detect Change/1
  Capacitance/1 -> Fault Inject/1(Nominal), Product1/2
  Product1/1 -> Integrator/3
  Integrator/1 -> Product2/1, q/1
  Reciprocal/1 -> Product2/2
  Fault Profile/1 -> Fault Inject/2(Fault Profile)
  Step/1 -> Fault Inject/3(Step)
  Ramp/1 -> Fault Inject/4(Ramp)
  Product2/1 -> E/1
  Detect Change/1 -> Data Type Conversion/1
  Data Type Conversion/1 -> Integrator/2
  Fault Inject/1(Out1) -> Saturation/1
  Saturation/1 -> Reciprocal/1, C/1

== system VirtualADAPT/VirtualADAPTv1/PowerStorage/Battery2Subsystem/Battery2/TemperatureModel/ModAmbTemp ==
  u(1) : Inport
  Function : Fcn  Expr=-u(1)
  Mux : Mux  Inputs=1
  y(1) : Outport
  wires:
  u(1)/1 -> Mux/1
  Mux/1 -> Function/1
  Function/1 -> y(1)/1

== system VirtualADAPT/VirtualADAPTv1/PowerStorage/Battery2Subsystem/Battery2/TemperatureModel/ModPs ==
  u(1) : Inport
  Function : Fcn  Expr=u(1)
  Mux : Mux  Inputs=1
  y(1) : Outport
  wires:
  u(1)/1 -> Mux/1
  Mux/1 -> Function/1
  Function/1 -> y(1)/1

== system VirtualADAPT/VirtualADAPTv1/PowerStorage/Battery2Subsystem/Battery2/TemperatureModel/OJ ==
  u(1) : Inport
  u(2) : Inport  Port=2
  u(3) : Inport  Port=3
  Adder : Sum  SaturateOnIntegerOverflow=on  InputSameDT=on  OutDataTypeStr=Inherit: Same as first input
  Gain : Gain  SaturateOnIntegerOverflow=on  OutDataTypeStr=Inherit: Same as input  ParamDataTypeStr=Inherit: Same as input
  y(1) : Outport
  y(2) : Outport  Port=2
  y(3) : Outport  Port=3
  O : Outport  Port=4
  wires:
  Adder/1 -> Gain/1
  u(2)/1 -> y(1)/1, y(3)/1, O/1
  u(1)/1 -> Adder/1
  Gain/1 -> y(2)/1
  u(3)/1 -> Adder/2

== system VirtualADAPT/VirtualADAPTv1/PowerStorage/Battery2Subsystem/Battery2/TemperatureModel/Rtheta ==
  In : Inport
  Bond : Constant  Value=2
  Computing R : Subsystem
  Fault Profile : Constant  Value=FP
  JuncDB : Constant  Value=2
  Junction Type : Constant
  Ramp : simulink/Sources/Ramp  slope=FM  start=FT  X0=R
  Resistor Value : Constant  Value=R
  Step : Step  Time=FT  Before=R  After=(1 + FM)*R  SampleTime=0
  Out : Outport
  R : Outport  Port=2
  wires:
  Computing R/1(Out1) -> Out/1
  In/1 -> Computing R/1(In1)
  Resistor Value/1 -> Computing R/2(RNominal)
  Fault Profile/1 -> Computing R/3(Fault Profile)
  Step/1 -> Computing R/4(Step)
  Ramp/1 -> Computing R/5(Ramp)
  JuncDB/1 -> Computing R/6(JuncDB)
  Bond/1 -> Computing R/7(Bond)
  Junction Type/1 -> Computing R/8(Junction Type)
  Computing R/2(Out2) -> R/1

== system VirtualADAPT/VirtualADAPTv1/PowerStorage/Battery2Subsystem/Battery2/TemperatureModel/SfPs ==
  E : Inport
  Sf : Inport  Port=2
  Bias : Step  Time=FT  After=FM  SampleTime=0
  Fault Inject : Subsystem
  Fault Profile : Constant  Value=FP
  Mult2 : Product  SaturateOnIntegerOverflow=on  RndMeth=Zero  InputSameDT=on  OutDataTypeStr=Inherit: Same as first input
  Multiply : Product  SaturateOnIntegerOverflow=on  RndMeth=Zero  InputSameDT=on  OutDataTypeStr=Inherit: Same as first input
  Ramp : simulink/Sources/Ramp  slope=FM  start=FT  X0=0
  Step : Step  Time=FT  Before=1  After=(1 + FM)  SampleTime=0
  StuckAt : Step  Time=FT  Before=1  After=0  SampleTime=0
  StuckAt2 : Step  Time=FT  After=FM  SampleTime=0
  Sum : Sum  SaturateOnIntegerOverflow=on  InputSameDT=on  OutDataTypeStr=Inherit: Same as first input
  Sum2 : Sum  SaturateOnIntegerOverflow=on  InputSameDT=on  OutDataTypeStr=Inherit: Same as first input
  Sum3 : Sum  SaturateOnIntegerOverflow=on  InputSameDT=on  OutDataTypeStr=Inherit: Same as first input
  F : Outport
  wires:
  Fault Inject/1(Out1) -> F/1
  Sf/1 -> Fault Inject/1(Nominal), Multiply/1, Sum/1, Sum2/1, Mult2/1
  Fault Profile/1 -> Fault Inject/2(Fault Profile)
  Step/1 -> Multiply/2
  Ramp/1 -> Sum/2
  Multiply/1 -> Fault Inject/3(Step)
  Sum/1 -> Fault Inject/4(Ramp)
  Bias/1 -> Sum2/2
  Sum2/1 -> Fault Inject/5(Bias)
  StuckAt/1 -> Mult2/2
  Mult2/1 -> Sum3/1
  StuckAt2/1 -> Sum3/2
  Sum3/1 -> Fault Inject/6(stuckAt)

== system VirtualADAPT/VirtualADAPTv1/PowerStorage/Battery2Subsystem/Battery2/TemperatureModel/theta ==
  u(1) : Inport
  u(2) : Inport  Port=2
  u(3) : Inport  Port=3
  Adder : Sum  Inputs=+-  SaturateOnIntegerOverflow=on  InputSameDT=on  OutDataTypeStr=Inherit: Same as first input
  Gain : Gain  SaturateOnIntegerOverflow=on  OutDataTypeStr=Inherit: Same as input  ParamDataTypeStr=Inherit: Same as input
  y(1) : Outport
  y(2) : Outport  Port=2
  y(3) : Outport  Port=3
  O : Outport  Port=4
  wires:
  Adder/1 -> Gain/1
  Gain/1 -> y(1)/1
  u(1)/1 -> y(2)/1, y(3)/1, O/1
  u(2)/1 -> Adder/1
  u(3)/1 -> Adder/2

== system VirtualADAPT/VirtualADAPTv1/PowerStorage/Battery2Subsystem/CircuitBreakerEY236/C/Fault Inject ==
  Nominal : Inport
  Fault Profile : Inport  Port=2
  Step : Inport  Port=3
  Ramp : Inport  Port=4
  Bias : Inport  Port=5
  stuckAt : Inport  Port=6
  Switch1 : MultiPortSwitch  Inputs=5  SaturateOnIntegerOverflow=on  InputSameDT=on
  Out1 : Outport
  wires:
  Nominal/1 -> Switch1/2
  Fault Profile/1 -> Switch1/1
  Step/1 -> Switch1/3
  Ramp/1 -> Switch1/4
  Bias/1 -> Switch1/5
  stuckAt/1 -> Switch1/6
  Switch1/1 -> Out1/1

== system VirtualADAPT/VirtualADAPTv1/PowerStorage/Battery2Subsystem/CircuitBreakerEY236/CurrentLimit/Fault Inject ==
  Nominal : Inport
  Fault Profile : Inport  Port=2
  Step : Inport  Port=3
  Ramp : Inport  Port=4
  Bias : Inport  Port=5
  stuckAt : Inport  Port=6
  Switch1 : MultiPortSwitch  Inputs=5  SaturateOnIntegerOverflow=on  InputSameDT=on
  Out1 : Outport
  wires:
  Nominal/1 -> Switch1/2
  Fault Profile/1 -> Switch1/1
  Step/1 -> Switch1/3
  Ramp/1 -> Switch1/4
  Bias/1 -> Switch1/5
  stuckAt/1 -> Switch1/6
  Switch1/1 -> Out1/1

== system VirtualADAPT/VirtualADAPTv1/PowerStorage/Battery2Subsystem/CircuitBreakerEY236/Delay/C ==
  F : Inport
  Capacitance : Constant  Value=C
  Fault Inject : Subsystem
  Fault Profile : Constant  Value=FP
  InitialCondition : Constant  Value=IC
  Integrator : Integrator  InitialConditionSource=external
  Product1 : Product  SaturateOnIntegerOverflow=on  RndMeth=Zero  InputSameDT=on  OutDataTypeStr=Inherit: Same as first input
  Product2 : Product  SaturateOnIntegerOverflow=on  RndMeth=Zero  InputSameDT=on  OutDataTypeStr=Inherit: Same as first input
  Ramp : simulink/Sources/Ramp  slope=FM  start=FT  X0=C
  Reciprocal : Math  Operator=reciprocal  SaturateOnIntegerOverflow=on
  Step : Step  Time=FT  Before=C  After=(1 + FM)*C  SampleTime=0
  E : Outport
  C : Outport  Port=2
  q : Outport  Port=3
  wires:
  F/1 -> Integrator/1
  InitialCondition/1 -> Product1/1
  Capacitance/1 -> Fault Inject/1(Nominal), Product1/2
  Product1/1 -> Integrator/2
  Integrator/1 -> Product2/1, q/1
  Fault Inject/1(Out1) -> Reciprocal/1, C/1
  Reciprocal/1 -> Product2/2
  Fault Profile/1 -> Fault Inject/2(Fault Profile)
  Step/1 -> Fault Inject/3(Step)
  Ramp/1 -> Fault Inject/4(Ramp)
  Product2/1 -> E/1

== system VirtualADAPT/VirtualADAPTv1/PowerStorage/Battery2Subsystem/CircuitBreakerEY236/Delay/Function ==
  u(1) : Inport
  Function : Fcn  Expr=u(1)
  Mux : Mux  Inputs=1
  y(1) : Outport
  wires:
  u(1)/1 -> Mux/1
  Mux/1 -> Function/1
  Function/1 -> y(1)/1

== system VirtualADAPT/VirtualADAPTv1/PowerStorage/Battery2Subsystem/CircuitBreakerEY236/Delay/OneJunction ==
  u(1) : Inport
  u(2) : Inport  Port=2
  u(3) : Inport  Port=3
  Adder : Sum  Inputs=+-  SaturateOnIntegerOverflow=on  InputSameDT=on  OutDataTypeStr=Inherit: Same as first input
  Gain : Gain  SaturateOnIntegerOverflow=on  OutDataTypeStr=Inherit: Same as input  ParamDataTypeStr=Inherit: Same as input
  y(1) : Outport
  y(2) : Outport  Port=2
  y(3) : Outport  Port=3
  O : Outport  Port=4
  wires:
  Adder/1 -> Gain/1
  Gain/1 -> y(1)/1
  u(1)/1 -> y(2)/1, y(3)/1, O/1
  u(2)/1 -> Adder/1
  u(3)/1 -> Adder/2

== system VirtualADAPT/VirtualADAPTv1/PowerStorage/Battery2Subsystem/CircuitBreakerEY236/Delay/R ==
  In : Inport
  Bond : Constant
  Computing R : Subsystem
  Fault Profile : Constant  Value=FP
  JuncDB : Constant
  Junction Type : Constant
  Ramp : simulink/Sources/Ramp  slope=FM  start=FT  X0=R
  Resistor Value : Constant  Value=R
  Step : Step  Time=FT  Before=R  After=(1 + FM)*R  SampleTime=0
  Out : Outport
  R : Outport  Port=2
  wires:
  Computing R/1(Out1) -> Out/1
  In/1 -> Computing R/1(In1)
  Resistor Value/1 -> Computing R/2(RNominal)
  Fault Profile/1 -> Computing R/3(Fault Profile)
  Step/1 -> Computing R/4(Step)
  Ramp/1 -> Computing R/5(Ramp)
  JuncDB/1 -> Computing R/6(JuncDB)
  Bond/1 -> Computing R/7(Bond)
  Junction Type/1 -> Computing R/8(Junction Type)
  Computing R/2(Out2) -> R/1

== system VirtualADAPT/VirtualADAPTv1/PowerStorage/Battery2Subsystem/CircuitBreakerEY236/Delay/Se ==
  F : Inport
  Se : Inport  Port=2
  Bias : Step  Time=FT  After=FM  SampleTime=0
  Fault Inject : Subsystem
  Fault Profile : Constant  Value=FP
  Mult2 : Product  SaturateOnIntegerOverflow=on  RndMeth=Zero  InputSameDT=on  OutDataTypeStr=Inherit: Same as first input
  Multiply : Product  SaturateOnIntegerOverflow=on  RndMeth=Zero  InputSameDT=on  OutDataTypeStr=Inherit: Same as first input
  Ramp : simulink/Sources/Ramp  slope=FM  start=FT  X0=0
  Step : Step  Time=FT  Before=1  After=(1 + FM)  SampleTime=0
  StuckAt : Step  Time=FT  Before=1  After=0  SampleTime=0
  StuckAt2 : Step  Time=FT  After=FM  SampleTime=0
  Sum : Sum  SaturateOnIntegerOverflow=on  InputSameDT=on  OutDataTypeStr=Inherit: Same as first input
  Sum2 : Sum  SaturateOnIntegerOverflow=on  InputSameDT=on  OutDataTypeStr=Inherit: Same as first input
  Sum3 : Sum  SaturateOnIntegerOverflow=on  InputSameDT=on  OutDataTypeStr=Inherit: Same as first input
  E : Outport
  wires:
  Fault Inject/1(Out1) -> E/1
  Se/1 -> Fault Inject/1(Nominal), Multiply/1, Sum/1, Sum2/1, Mult2/1
  Fault Profile/1 -> Fault Inject/2(Fault Profile)
  Step/1 -> Multiply/2
  Ramp/1 -> Sum/2
  Multiply/1 -> Fault Inject/3(Step)
  Sum/1 -> Fault Inject/4(Ramp)
  Bias/1 -> Sum2/2
  Sum2/1 -> Fault Inject/5(Bias)
  StuckAt/1 -> Mult2/2
  Mult2/1 -> Sum3/1
  StuckAt2/1 -> Sum3/2
  Sum3/1 -> Fault Inject/6(stuckAt)

== system VirtualADAPT/VirtualADAPTv1/PowerStorage/Battery2Subsystem/CircuitBreakerEY236/Delay/ZeroJunction ==
  u(1) : Inport
  u(2) : Inport  Port=2
  Adder : Sum  Inputs=+  SaturateOnIntegerOverflow=on  InputSameDT=on  OutDataTypeStr=Inherit: Same as first input
  Gain : Gain  SaturateOnIntegerOverflow=on  OutDataTypeStr=Inherit: Same as input  ParamDataTypeStr=Inherit: Same as input
  y(1) : Outport
  y(2) : Outport  Port=2
  O : Outport  Port=3
  wires:
  Adder/1 -> Gain/1
  Gain/1 -> y(1)/1
  u(1)/1 -> y(2)/1, O/1
  u(2)/1 -> Adder/1

== system VirtualADAPT/VirtualADAPTv1/PowerStorage/Battery2Subsystem/CircuitBreakerEY236/ParallelConn/determineState ==
  In1 : Inport
  In2 : Inport  Port=2
  Fcn : Fcn  Expr=u(2) && u(3)
  Memory : Memory  X0=1
  Mux : Mux  Inputs=3
  Out1 : Outport
  wires:
  Memory/1 -> Mux/1
  In1/1 -> Mux/2
  In2/1 -> Mux/3
  Mux/1 -> Fcn/1
  Fcn/1 -> Memory/1, Out1/1

== system VirtualADAPT/VirtualADAPTv1/PowerStorage/Battery2Subsystem/CircuitBreakerEY236/R/Computing R ==
  In1 : Inport
  RNominal : Inport  Port=2
  Fault Profile : Inport  Port=3
  Step : Inport  Port=4
  Ramp : Inport  Port=5
  JuncDB : Inport  Port=6
  Bond : Inport  Port=7
  Junction Type : Inport  Port=8
  CalcCausality : Fcn  Expr=(u(1) == u(2) && u(3) ==1) || (u(1) !=u(2) && u(3) == 0)
  CalcOutput : Fcn  Expr=(u(1)*u(2)^u(3))
  MinusOne : Constant  Value=-1
  Mux1 : Mux  Inputs=3
  Mux2 : Mux  Inputs=3
  One : Constant
  Switch1 : MultiPortSwitch  SaturateOnIntegerOverflow=on  InputSameDT=on
  Switch2 : MultiPortSwitch  DataPortOrder=Zero-based contiguous  Inputs=2  zeroidx=on  SaturateOnIntegerOverflow=on  InputSameDT=on
  Out1 : Outport
  Out2 : Outport  Port=2
  wires:
  In1/1 -> Mux2/1
  Fault Profile/1 -> Switch1/1
  RNominal/1 -> Switch1/2
  Step/1 -> Switch1/3
  Ramp/1 -> Switch1/4
  Switch1/1 -> Out2/1, Mux2/2
  JuncDB/1 -> Mux1/1
  Bond/1 -> Mux1/2
  Junction Type/1 -> Mux1/3
  Mux1/1 -> CalcCausality/1
  CalcCausality/1 -> Switch2/1
  One/1 -> Switch2/2
  MinusOne/1 -> Switch2/3
  Switch2/1 -> Mux2/3
  Mux2/1 -> CalcOutput/1
  CalcOutput/1 -> Out1/1

== system VirtualADAPT/VirtualADAPTv1/PowerStorage/Battery2Subsystem/RelayEY241/ParallelConn/determineState ==
  In1 : Inport
  In2 : Inport  Port=2
  Fcn : Fcn  Expr=(!u(1)&&u(2)) || (u(1)&&(!u(3)))
  Memory : Memory
  Mux : Mux  Inputs=3
  Out1 : Outport
  wires:
  Memory/1 -> Mux/1
  In1/1 -> Mux/2
  In2/1 -> Mux/3
  Mux/1 -> Fcn/1
  Fcn/1 -> Memory/1, Out1/1

== system VirtualADAPT/VirtualADAPTv1/PowerStorage/Battery2Subsystem/RelayEY241/Relay/determineState ==
  In1 : Inport
  In2 : Inport  Port=2
  Fcn : Fcn  Expr=(!u(1)&&u(2)) || (u(1)&&(!u(3)))
  Memory : Memory
  Mux : Mux  Inputs=3
  Out1 : Outport
  wires:
  Memory/1 -> Mux/1
  In1/1 -> Mux/2
  In2/1 -> Mux/3
  Mux/1 -> Fcn/1
  Fcn/1 -> Memory/1, Out1/1

== system VirtualADAPT/VirtualADAPTv1/PowerStorage/Battery2Subsystem/RelayEY241/Sensor/MSe ==
  F : Inport
  Se : Inport  Port=2
  Bias : Step  Time=FT  After=FM  SampleTime=0
  Fault Inject : Subsystem
  Fault Profile : Constant  Value=FP
  Mult2 : Product  SaturateOnIntegerOverflow=on  RndMeth=Zero  InputSameDT=on  OutDataTypeStr=Inherit: Same as first input
  Multiply : Product  SaturateOnIntegerOverflow=on  RndMeth=Zero  InputSameDT=on  OutDataTypeStr=Inherit: Same as first input
  Ramp : simulink/Sources/Ramp  slope=FM  start=FT  X0=0
  Step : Step  Time=FT  Before=1  After=(1 + FM)  SampleTime=0
  StuckAt : Step  Time=FT  Before=1  After=0  SampleTime=0
  StuckAt2 : Step  Time=FT  After=FM  SampleTime=0
  Sum : Sum  SaturateOnIntegerOverflow=on  InputSameDT=on  OutDataTypeStr=Inherit: Same as first input
  Sum2 : Sum  SaturateOnIntegerOverflow=on  InputSameDT=on  OutDataTypeStr=Inherit: Same as first input
  Sum3 : Sum  SaturateOnIntegerOverflow=on  InputSameDT=on  OutDataTypeStr=Inherit: Same as first input
  E : Outport
  wires:
  Fault Inject/1(Out1) -> E/1
  Se/1 -> Fault Inject/1(Nominal), Multiply/1, Sum/1, Sum2/1, Mult2/1
  Fault Profile/1 -> Fault Inject/2(Fault Profile)
  Step/1 -> Multiply/2
  Ramp/1 -> Sum/2
  Multiply/1 -> Fault Inject/3(Step)
  Sum/1 -> Fault Inject/4(Ramp)
  Bias/1 -> Sum2/2
  Sum2/1 -> Fault Inject/5(Bias)
  StuckAt/1 -> Mult2/2
  Mult2/1 -> Sum3/1
  StuckAt2/1 -> Sum3/2
  Sum3/1 -> Fault Inject/6(stuckAt)
